simulink model "TSR_Scenario_005_cs"  (Simulink 7.6) (continued)
== system TSR_Scenario_005_cs == (continued)
      mask help:
      | The Actor State contains a vector signal of size (9x1), containing the following signals:
      |
      | -  Position                  (x,y,z)                                     [m]
      | -  Velocity                  (vx,vy,vz)                               [m/s]
      | -  Angles                    (Roll, Pitch, Yaw)                   [rad]
      |
      | The Actor state is defined in the global axis system.
      | The state is superponed on the Initial position defined in the GUI.
      |
      | For more information, please click Help.
      settings: DeleteFcn=PreScanBlockDeleteFcn;
      mask Help: eval('openHelp(222)')
      mask Display: image(imread('State.png'))
  STATE_Toyota_Previa_2 : Subsystem  Tag=State  Priority=0  mask(PreScan Sink Block: State){x0=115.06995391845703, y0=52.373069763183594, z0=0.70999997854232788, Roll0=0, Pitch0=0, Yaw0=0, TimeStep=1/100}
      mask prompts: x0 "Ini x-position VEHICLE wrt GLOBAL axis system [m]"; y0 "Ini y-position VEHICLE wrt GLOBAL axis system [m]"; z0 "Ini z-position VEHICLE wrt GLOBAL axis system [m]"; Roll0 "Ini roll-angle BODY wrt VEHICLE axis system [deg]"; Pitch0 "Ini pitch-angle BODY wrt VEHICLE axis system [deg]"; Yaw0 "Ini yaw-angle VEHICLE wrt GLOBAL axis system [deg]"; TimeStep "Time Step [s]"
      mask help:
      | The Actor State contains a vector signal of size (9x1), containing the following signals:
      |
      | -  Position                  (x,y,z)                                     [m]
      | -  Velocity                  (vx,vy,vz)                               [m/s]
      | -  Angles                    (Roll, Pitch, Yaw)                   [rad]
      |
      | The Actor state is defined in the global axis system.
      | The state is superponed on the Initial position defined in the GUI.
      |
      | For more information, please click Help.
      settings: DeleteFcn=PreScanBlockDeleteFcn;
      mask Help: eval('openHelp(222)')
      mask Display: image(imread('State.png'))
  STATE_Toyota_Previa_3 : Subsystem  Tag=State  Priority=0  mask(PreScan Sink Block: State){x0=94.289756774902344, y0=52.638362884521484, z0=0.70999997854232788, Roll0=0, Pitch0=0, Yaw0=0, TimeStep=1/100}
      mask prompts: x0 "Ini x-position VEHICLE wrt GLOBAL axis system [m]"; y0 "Ini y-position VEHICLE wrt GLOBAL axis system [m]"; z0 "Ini z-position VEHICLE wrt GLOBAL axis system [m]"; Roll0 "Ini roll-angle BODY wrt VEHICLE axis system [deg]"; Pitch0 "Ini pitch-angle BODY wrt VEHICLE axis system [deg]"; Yaw0 "Ini yaw-angle VEHICLE wrt GLOBAL axis system [deg]"; TimeStep "Time Step [s]"
      mask help:
      | The Actor State contains a vector signal of size (9x1), containing the following signals:
      |
      | -  Position                  (x,y,z)                                     [m]
      | -  Velocity                  (vx,vy,vz)                               [m/s]
      | -  Angles                    (Roll, Pitch, Yaw)                   [rad]
      |
      | The Actor state is defined in the global axis system.
      | The state is superponed on the Initial position defined in the GUI.
      |
      | For more information, please click Help.
      settings: DeleteFcn=PreScanBlockDeleteFcn;
      mask Help: eval('openHelp(222)')
      mask Display: image(imread('State.png'))
  STATE_Toyota_Previa_4 : Subsystem  Tag=State  Priority=0  mask(PreScan Sink Block: State){x0=109.62726593017578, y0=90.440689086914063, z0=0.70999997854232788, Roll0=0, Pitch0=0, Yaw0=90, TimeStep=1/100}
      mask prompts: x0 "Ini x-position VEHICLE wrt GLOBAL axis system [m]"; y0 "Ini y-position VEHICLE wrt GLOBAL axis system [m]"; z0 "Ini z-position VEHICLE wrt GLOBAL axis system [m]"; Roll0 "Ini roll-angle BODY wrt VEHICLE axis system [deg]"; Pitch0 "Ini pitch-angle BODY wrt VEHICLE axis system [deg]"; Yaw0 "Ini yaw-angle VEHICLE wrt GLOBAL axis system [deg]"; TimeStep "Time Step [s]"
      mask help:
      | The Actor State contains a vector signal of size (9x1), containing the following signals:
      |
      | -  Position                  (x,y,z)                                     [m]
      | -  Velocity                  (vx,vy,vz)                               [m/s]
      | -  Angles                    (Roll, Pitch, Yaw)                   [rad]
      |
      | The Actor state is defined in the global axis system.
      | The state is superponed on the Initial position defined in the GUI.
      |
      | For more information, please click Help.
      settings: DeleteFcn=PreScanBlockDeleteFcn;
      mask Help: eval('openHelp(222)')
      mask Display: image(imread('State.png'))
  STATE_Toyota_Yaris_1 : Subsystem  Tag=State  Priority=0  mask(PreScan Sink Block: State){x0=144.99999618530273, y0=63.161239624023438, z0=0.57, Roll0=0, Pitch0=0, Yaw0=180, TimeStep=1/100}
      mask prompts: x0 "Ini x-position VEHICLE wrt GLOBAL axis system [m]"; y0 "Ini y-position VEHICLE wrt GLOBAL axis system [m]"; z0 "Ini z-position VEHICLE wrt GLOBAL axis system [m]"; Roll0 "Ini roll-angle BODY wrt VEHICLE axis system [deg]"; Pitch0 "Ini pitch-angle BODY wrt VEHICLE axis system [deg]"; Yaw0 "Ini yaw-angle VEHICLE wrt GLOBAL axis system [deg]"; TimeStep "Time Step [s]"
      mask help:
      | The Actor State contains a vector signal of size (9x1), containing the following signals:
      |
      | -  Position                  (x,y,z)                                     [m]
      | -  Velocity                  (vx,vy,vz)                               [m/s]
      | -  Angles                    (Roll, Pitch, Yaw)                   [rad]
      |
      | The Actor state is defined in the global axis system.
      | The state is superponed on the Initial position defined in the GUI.
      |
      | For more information, please click Help.
      settings: DeleteFcn=PreScanBlockDeleteFcn;
      mask Help: eval('openHelp(222)')
      mask Display: image(imread('State.png'))
  STATE_Toyota_Yaris_2 : Subsystem  Tag=State  Priority=0  mask(PreScan Sink Block: State){x0=318.75335693359375, y0=147.367919921875, z0=0.57, Roll0=0, Pitch0=0, Yaw0=0, TimeStep=1/100}
      mask prompts: x0 "Ini x-position VEHICLE wrt GLOBAL axis system [m]"; y0 "Ini y-position VEHICLE wrt GLOBAL axis system [m]"; z0 "Ini z-position VEHICLE wrt GLOBAL axis system [m]"; Roll0 "Ini roll-angle BODY wrt VEHICLE axis system [deg]"; Pitch0 "Ini pitch-angle BODY wrt VEHICLE axis system [deg]"; Yaw0 "Ini yaw-angle VEHICLE wrt GLOBAL axis system [deg]"; TimeStep "Time Step [s]"
      mask help:
      | The Actor State contains a vector signal of size (9x1), containing the following signals:
      |
      | -  Position                  (x,y,z)                                     [m]
      | -  Velocity                  (vx,vy,vz)                               [m/s]
      | -  Angles                    (Roll, Pitch, Yaw)                   [rad]
      |
      | The Actor state is defined in the global axis system.
      | The state is superponed on the Initial position defined in the GUI.
      |
      | For more information, please click Help.
      settings: DeleteFcn=PreScanBlockDeleteFcn;
      mask Help: eval('openHelp(222)')
      mask Display: image(imread('State.png'))
  SetActuators : Subsystem  Tag=SetActuators  Priority=1  mask(PreScan Simulation Core Block){TimeStep=1/100, NrOfActuators=1661}
      mask prompts: TimeStep "Timestep [s]"; NrOfActuators "Number of actuator values"
      mask help:
      | Please do not remove this block from the PreScan compilation sheet,
      | as it contains essential configuration parameters for a PreScan simulation.
      settings: DeleteFcn=PreScanBlockDeleteFcn;
  SimCoreConfiguration : M-S-Function  Tag=SimCoreConfiguration  Description=128EA0B91E8905FD20CD9FF5500624459A08174AB169DD15EB1AFE5773DF0866  FunctionName=SimCoreBlock  Parameters=ExperimentXML PortsXML SimulationCore HostName CheckStart EnableLog RfR EnableGPUAccel ShowComputationTime  mask(PreScan Simulation Core Configurator){SimulationCore=NUTS, HostName=0, CheckStart=0, prescanversion='7.4.0', EnableLog=off, RfR=0, EnableGPUAccel=off, ShowComputationTime=off}
      mask prompts: SimulationCore "Simulation Core"; HostName "Simulation Host"; CheckStart "Show START Dialog"; prescanversion "PreScan Version"; EnableLog "Create TA Log"; RfR "Record for Replay"; EnableGPUAccel "Enable GPU Acceleration"; ShowComputationTime "Show ComputationTime Scope"
      mask help:
      | Please do not remove this block from the PreScan compilation sheet,
      | as it contains essential configuration parameter for a PreScan simulation.
      mask init:
      | model = bdroot;
      | ExperimentXML = [model(1:end-3) '.pex'];
      | PortsXML = [model '_ports.pcd'];
      settings: DeleteFcn=PreScanBlockDeleteFcn;  ModelCloseFcn=assignin('base',['model_closed_' bdroot],true);
      mask Help: eval('openHelp(812)')
      mask Display: image(imread('prescanlogo.ico'))
  SimCoreInfo : Subsystem  Tag=SimCoreInfo
  Sphere_1 : Subsystem  Tag=WorldObjectSubSystem  InitFcn=UniqueId = '237';
      mask Display: image(imread('Sphere.jpg'));color('white');text(0,0,' ID=68\n')
  Toyota_Previa_1 : Subsystem  Tag=WorldObjectSubSystem  InitFcn=UniqueId = '24';
      mask Display: image(imread('Toyota_Previa.jpg'));color('white');text(0,0,' ID=11\n')
  Toyota_Previa_2 : Subsystem  Tag=WorldObjectSubSystem  InitFcn=UniqueId = '141';
      mask Display: image(imread('Toyota_Previa.jpg'));color('white');text(0,0,' ID=47\n')
  Toyota_Previa_3 : Subsystem  Tag=WorldObjectSubSystem  InitFcn=UniqueId = '142';
      mask Display: image(imread('Toyota_Previa.jpg'));color('white');text(0,0,' ID=48\n')
  Toyota_Previa_4 : Subsystem  Tag=WorldObjectSubSystem  InitFcn=UniqueId = '164';
      mask Display: image(imread('Toyota_Previa.jpg'));color('white');text(0,0,' ID=93\n')
  Toyota_Yaris_1 : Subsystem  Tag=WorldObjectSubSystem  InitFcn=UniqueId = '225';
      mask Display: image(imread('Toyota_Yaris.jpg'));color('white');text(0,0,' ID=32\n')
  Toyota_Yaris_2 : Subsystem  Tag=WorldObjectSubSystem  InitFcn=UniqueId = '610';
      mask Display: image(imread('Toyota_Yaris.jpg'));color('white');text(0,0,' ID=222\n')
  TrafficLightRoadSideNL_1 : Subsystem  Tag=WorldObjectSubSystem  InitFcn=UniqueId = '664';
      mask Display: image(imread('TrafficLightRoadSideNL.jpg'));color('white');text(0,0,' ID=235\n')
  TrafficLightRoadSideNL_2 : Subsystem  Tag=WorldObjectSubSystem  InitFcn=UniqueId = '665';
      mask Display: image(imread('TrafficLightRoadSideNL.jpg'));color('white');text(0,0,' ID=273\n')
  TrafficLightRoadSideNL_3 : Subsystem  Tag=WorldObjectSubSystem  InitFcn=UniqueId = '666';
      mask Display: image(imread('TrafficLightRoadSideNL.jpg'));color('white');text(0,0,' ID=274\n')
  TrafficLightRoadSideNL_4 : Subsystem  Tag=WorldObjectSubSystem  InitFcn=UniqueId = '667';
      mask Display: image(imread('TrafficLightRoadSideNL.jpg'));color('white');text(0,0,' ID=275\n')
  TrafficLightRoadSideNL_5 : Subsystem  Tag=WorldObjectSubSystem  InitFcn=UniqueId = '668';
      mask Display: image(imread('TrafficLightRoadSideNL.jpg'));color('white');text(0,0,' ID=276\n')
  TrafficLightRoadSideNL_6 : Subsystem  Tag=WorldObjectSubSystem  InitFcn=UniqueId = '669';
      mask Display: image(imread('TrafficLightRoadSideNL.jpg'));color('white');text(0,0,' ID=277\n')
  TrafficLightRoadSideNL_7 : Subsystem  Tag=WorldObjectSubSystem  InitFcn=UniqueId = '670';
      mask Display: image(imread('TrafficLightRoadSideNL.jpg'));color('white');text(0,0,' ID=278\n')
  TrafficLightRoadSideNL_8 : Subsystem  Tag=WorldObjectSubSystem  InitFcn=UniqueId = '671';
      mask Display: image(imread('TrafficLightRoadSideNL.jpg'));color('white');text(0,0,' ID=279\n')
  VISUBrakeLightL_Audi_A8_1 : Subsystem  Tag=VisuLights  Priority=0
      settings: DeleteFcn=PreScanBlockDeleteFcn;
      mask Type: PreScan Sink Block: VisuLights
      mask Description:
      | The VisuLights block provides a way to animate the active lights of a vehicle with possibilities to adapt the:
      | - Intensity [-]
      | - Position (x,y,z) [m]
      | - Orientation (Roll, Pitch, Yaw) [deg]
      | - Color (RGB) [-]
      | - TextureResourceID [-]
      | 
      | For more information, please click Help.
      mask Help: eval('openHelp(231)')
      mask Display: image(imread('HeadLight.png'))
  VISUBrakeLightR_Audi_A8_1 : Subsystem  Tag=VisuLights  Priority=0
      settings: DeleteFcn=PreScanBlockDeleteFcn;
      mask Type: PreScan Sink Block: VisuLights
      mask Description:
      | The VisuLights block provides a way to animate the active lights of a vehicle with possibilities to adapt the:
      | - Intensity [-]
      | - Position (x,y,z) [m]
      | - Orientation (Roll, Pitch, Yaw) [deg]
      | - Color (RGB) [-]
      | - TextureResourceID [-]
      | 
      | For more information, please click Help.
      mask Help: eval('openHelp(231)')
      mask Display: image(imread('HeadLight.png'))
  VISUCasingLights_TrafficLightRoadSideNL_1 : Subsystem  Tag=VisuCasingLights  Priority=0
      settings: DeleteFcn=PreScanBlockDeleteFcn;
      mask Type: PreScan Sink Block: VisuLights
      mask Description:
      | The VisuLights block provides a way to animate the indicator and braking lights of a vehicle.
      | 
      | For more information, please click Help.
      mask Help: eval('openHelp(223)')
      mask Display: image(imread('TrafficLightCasing.png'))
  VISUCasingLights_TrafficLightRoadSideNL_2 : Subsystem  Tag=VisuCasingLights  Priority=0
      settings: DeleteFcn=PreScanBlockDeleteFcn;
      mask Type: PreScan Sink Block: VisuLights
      mask Description:
      | The VisuLights block provides a way to animate the indicator and braking lights of a vehicle.
      | 
      | For more information, please click Help.
      mask Help: eval('openHelp(223)')
      mask Display: image(imread('TrafficLightCasing.png'))
  VISUCasingLights_TrafficLightRoadSideNL_3 : Subsystem  Tag=VisuCasingLights  Priority=0
      settings: DeleteFcn=PreScanBlockDeleteFcn;
      mask Type: PreScan Sink Block: VisuLights
      mask Description:
      | The VisuLights block provides a way to animate the indicator and braking lights of a vehicle.
      | 
      | For more information, please click Help.
      mask Help: eval('openHelp(223)')
      mask Display: image(imread('TrafficLightCasing.png'))
  VISUCasingLights_TrafficLightRoadSideNL_4 : Subsystem  Tag=VisuCasingLights  Priority=0
      settings: DeleteFcn=PreScanBlockDeleteFcn;
      mask Type: PreScan Sink Block: VisuLights
      mask Description:
      | The VisuLights block provides a way to animate the indicator and braking lights of a vehicle.
      | 
      | For more information, please click Help.
      mask Help: eval('openHelp(223)')
      mask Display: image(imread('TrafficLightCasing.png'))
  VISUCasingLights_TrafficLightRoadSideNL_5 : Subsystem  Tag=VisuCasingLights  Priority=0
      settings: DeleteFcn=PreScanBlockDeleteFcn;
      mask Type: PreScan Sink Block: VisuLights
      mask Description:
      | The VisuLights block provides a way to animate the indicator and braking lights of a vehicle.
      | 
      | For more information, please click Help.
      mask Help: eval('openHelp(223)')
      mask Display: image(imread('TrafficLightCasing.png'))
  VISUCasingLights_TrafficLightRoadSideNL_6 : Subsystem  Tag=VisuCasingLights  Priority=0
      settings: DeleteFcn=PreScanBlockDeleteFcn;
      mask Type: PreScan Sink Block: VisuLights
      mask Description:
      | The VisuLights block provides a way to animate the indicator and braking lights of a vehicle.
      | 
      | For more information, please click Help.
      mask Help: eval('openHelp(223)')
      mask Display: image(imread('TrafficLightCasing.png'))
  VISUCasingLights_TrafficLightRoadSideNL_7 : Subsystem  Tag=VisuCasingLights  Priority=0
      settings: DeleteFcn=PreScanBlockDeleteFcn;
      mask Type: PreScan Sink Block: VisuLights
      mask Description:
      | The VisuLights block provides a way to animate the indicator and braking lights of a vehicle.
      | 
      | For more information, please click Help.
      mask Help: eval('openHelp(223)')
      mask Display: image(imread('TrafficLightCasing.png'))
  VISUCasingLights_TrafficLightRoadSideNL_8 : Subsystem  Tag=VisuCasingLights  Priority=0
      settings: DeleteFcn=PreScanBlockDeleteFcn;
      mask Type: PreScan Sink Block: VisuLights
      mask Description:
      | The VisuLights block provides a way to animate the indicator and braking lights of a vehicle.
      | 
      | For more information, please click Help.
      mask Help: eval('openHelp(223)')
      mask Display: image(imread('TrafficLightCasing.png'))
  VISUHeadLightLeftHBLB_Audi_A8_1 : Subsystem  Tag=VisuLights  Priority=0
      settings: DeleteFcn=PreScanBlockDeleteFcn;
      mask Type: PreScan Sink Block: VisuLights
      mask Description:
      | The VisuLights block provides a way to animate the active lights of a vehicle with possibilities to adapt the:
      | - Intensity [-]
      | - Position (x,y,z) [m]
      | - Orientation (Roll, Pitch, Yaw) [deg]
      | - Color (RGB) [-]
      | - TextureResourceID [-]
      | 
      | For more information, please click Help.
      mask Help: eval('openHelp(231)')
      mask Display: image(imread('HeadLight.png'))
  VISUHeadLightRightHBLB_Audi_A8_1 : Subsystem  Tag=VisuLights  Priority=0
      settings: DeleteFcn=PreScanBlockDeleteFcn;
      mask Type: PreScan Sink Block: VisuLights
      mask Description:
      | The VisuLights block provides a way to animate the active lights of a vehicle with possibilities to adapt the:
      | - Intensity [-]
      | - Position (x,y,z) [m]
      | - Orientation (Roll, Pitch, Yaw) [deg]
      | - Color (RGB) [-]
      | - TextureResourceID [-]
      | 
      | For more information, please click Help.
      mask Help: eval('openHelp(231)')
      mask Display: image(imread('HeadLight.png'))
  VISULightPostLight_Lightpost_1 : Subsystem  Tag=VisuLights  Priority=0
      settings: DeleteFcn=PreScanBlockDeleteFcn;
      mask Type: PreScan Sink Block: VisuLights
      mask Description:
      | The VisuLights block provides a way to animate the active lights of a vehicle with possibilities to adapt the:
      | - Intensity [-]
      | - Position (x,y,z) [m]
      | - Orientation (Roll, Pitch, Yaw) [deg]
      | - Color (RGB) [-]
      | - TextureResourceID [-]
      | 
      | For more information, please click Help.
      mask Help: eval('openHelp(231)')
      mask Display: image(imread('HeadLight.png'))
  VISULightPostLight_Lightpost_11 : Subsystem  Tag=VisuLights  Priority=0
      settings: DeleteFcn=PreScanBlockDeleteFcn;
      mask Type: PreScan Sink Block: VisuLights
      mask Description:
      | The VisuLights block provides a way to animate the active lights of a vehicle with possibilities to adapt the:
      | - Intensity [-]
      | - Position (x,y,z) [m]
      | - Orientation (Roll, Pitch, Yaw) [deg]
      | - Color (RGB) [-]
      | - TextureResourceID [-]
      | 
      | For more information, please click Help.
      mask Help: eval('openHelp(231)')
      mask Display: image(imread('HeadLight.png'))
  VISULightPostLight_Lightpost_12 : Subsystem  Tag=VisuLights  Priority=0
      settings: DeleteFcn=PreScanBlockDeleteFcn;
      mask Type: PreScan Sink Block: VisuLights
      mask Description:
      | The VisuLights block provides a way to animate the active lights of a vehicle with possibilities to adapt the:
      | - Intensity [-]
      | - Position (x,y,z) [m]
      | - Orientation (Roll, Pitch, Yaw) [deg]
      | - Color (RGB) [-]
      | - TextureResourceID [-]
      | 
      | For more information, please click Help.
      mask Help: eval('openHelp(231)')
      mask Display: image(imread('HeadLight.png'))
  VISULightPostLight_Lightpost_2 : Subsystem  Tag=VisuLights  Priority=0
      settings: DeleteFcn=PreScanBlockDeleteFcn;
      mask Type: PreScan Sink Block: VisuLights
      mask Description:
      | The VisuLights block provides a way to animate the active lights of a vehicle with possibilities to adapt the:
      | - Intensity [-]
      | - Position (x,y,z) [m]
      | - Orientation (Roll, Pitch, Yaw) [deg]
      | - Color (RGB) [-]
      | - TextureResourceID [-]
      | 
      | For more information, please click Help.
      mask Help: eval('openHelp(231)')
      mask Display: image(imread('HeadLight.png'))
  VISULightPostLight_Lightpost_25 : Subsystem  Tag=VisuLights  Priority=0
      settings: DeleteFcn=PreScanBlockDeleteFcn;
      mask Type: PreScan Sink Block: VisuLights
      mask Description:
      | The VisuLights block provides a way to animate the active lights of a vehicle with possibilities to adapt the:
      | - Intensity [-]
      | - Position (x,y,z) [m]
      | - Orientation (Roll, Pitch, Yaw) [deg]
      | - Color (RGB) [-]
      | - TextureResourceID [-]
      | 
      | For more information, please click Help.
      mask Help: eval('openHelp(231)')
      mask Display: image(imread('HeadLight.png'))
  VISULightPostLight_Lightpost_27 : Subsystem  Tag=VisuLights  Priority=0
      settings: DeleteFcn=PreScanBlockDeleteFcn;
      mask Type: PreScan Sink Block: VisuLights
      mask Description:
      | The VisuLights block provides a way to animate the active lights of a vehicle with possibilities to adapt the:
      | - Intensity [-]
      | - Position (x,y,z) [m]
      | - Orientation (Roll, Pitch, Yaw) [deg]
      | - Color (RGB) [-]
      | - TextureResourceID [-]
      | 
      | For more information, please click Help.
      mask Help: eval('openHelp(231)')
      mask Display: image(imread('HeadLight.png'))
  VISULightPostLight_Lightpost_3 : Subsystem  Tag=VisuLights  Priority=0
      settings: DeleteFcn=PreScanBlockDeleteFcn;
      mask Type: PreScan Sink Block: VisuLights
      mask Description:
      | The VisuLights block provides a way to animate the active lights of a vehicle with possibilities to adapt the:
      | - Intensity [-]
      | - Position (x,y,z) [m]
      | - Orientation (Roll, Pitch, Yaw) [deg]
      | - Color (RGB) [-]
      | - TextureResourceID [-]
      | 
      | For more information, please click Help.
      mask Help: eval('openHelp(231)')
      mask Display: image(imread('HeadLight.png'))
  VISULightPostLight_Lightpost_34 : Subsystem  Tag=VisuLights  Priority=0
      settings: DeleteFcn=PreScanBlockDeleteFcn;
      mask Type: PreScan Sink Block: VisuLights
      mask Description:
      | The VisuLights block provides a way to animate the active lights of a vehicle with possibilities to adapt the:
      | - Intensity [-]
      | - Position (x,y,z) [m]
      | - Orientation (Roll, Pitch, Yaw) [deg]
      | - Color (RGB) [-]
      | - TextureResourceID [-]
      | 
      | For more information, please click Help.
      mask Help: eval('openHelp(231)')
      mask Display: image(imread('HeadLight.png'))
  VISULightPostLight_Lightpost_36 : Subsystem  Tag=VisuLights  Priority=0
      settings: DeleteFcn=PreScanBlockDeleteFcn;
      mask Type: PreScan Sink Block: VisuLights
      mask Description:
      | The VisuLights block provides a way to animate the active lights of a vehicle with possibilities to adapt the:
      | - Intensity [-]
      | - Position (x,y,z) [m]
      | - Orientation (Roll, Pitch, Yaw) [deg]
      | - Color (RGB) [-]
      | - TextureResourceID [-]
      | 
      | For more information, please click Help.
      mask Help: eval('openHelp(231)')
      mask Display: image(imread('HeadLight.png'))
  VISULightPostLight_Lightpost_38 : Subsystem  Tag=VisuLights  Priority=0
      settings: DeleteFcn=PreScanBlockDeleteFcn;
      mask Type: PreScan Sink Block: VisuLights
      mask Description:
      | The VisuLights block provides a way to animate the active lights of a vehicle with possibilities to adapt the:
      | - Intensity [-]
      | - Position (x,y,z) [m]
      | - Orientation (Roll, Pitch, Yaw) [deg]
      | - Color (RGB) [-]
      | - TextureResourceID [-]
      | 
      | For more information, please click Help.
      mask Help: eval('openHelp(231)')
      mask Display: image(imread('HeadLight.png'))
  VISULightPostLight_Lightpost_4 : Subsystem  Tag=VisuLights  Priority=0
      settings: DeleteFcn=PreScanBlockDeleteFcn;
      mask Type: PreScan Sink Block: VisuLights
      mask Description:
      | The VisuLights block provides a way to animate the active lights of a vehicle with possibilities to adapt the:
      | - Intensity [-]
      | - Position (x,y,z) [m]
      | - Orientation (Roll, Pitch, Yaw) [deg]
      | - Color (RGB) [-]
      | - TextureResourceID [-]
      | 
      | For more information, please click Help.
      mask Help: eval('openHelp(231)')
      mask Display: image(imread('HeadLight.png'))
  VISULightPostLight_Lightpost_40 : Subsystem  Tag=VisuLights  Priority=0
      settings: DeleteFcn=PreScanBlockDeleteFcn;
      mask Type: PreScan Sink Block: VisuLights
      mask Description:
      | The VisuLights block provides a way to animate the active lights of a vehicle with possibilities to adapt the:
      | - Intensity [-]
      | - Position (x,y,z) [m]
      | - Orientation (Roll, Pitch, Yaw) [deg]
      | - Color (RGB) [-]
      | - TextureResourceID [-]
      | 
      | For more information, please click Help.
      mask Help: eval('openHelp(231)')
      mask Display: image(imread('HeadLight.png'))
  VISULightPostLight_Lightpost_42 : Subsystem  Tag=VisuLights  Priority=0
      settings: DeleteFcn=PreScanBlockDeleteFcn;
      mask Type: PreScan Sink Block: VisuLights
      mask Description:
      | The VisuLights block provides a way to animate the active lights of a vehicle with possibilities to adapt the:
      | - Intensity [-]
      | - Position (x,y,z) [m]
      | - Orientation (Roll, Pitch, Yaw) [deg]
      | - Color (RGB) [-]
      | - TextureResourceID [-]
      | 
      | For more information, please click Help.
      mask Help: eval('openHelp(231)')
      mask Display: image(imread('HeadLight.png'))
  VISULightPostLight_Lightpost_44 : Subsystem  Tag=VisuLights  Priority=0
      settings: DeleteFcn=PreScanBlockDeleteFcn;
      mask Type: PreScan Sink Block: VisuLights
      mask Description:
      | The VisuLights block provides a way to animate the active lights of a vehicle with possibilities to adapt the:
      | - Intensity [-]
      | - Position (x,y,z) [m]
      | - Orientation (Roll, Pitch, Yaw) [deg]
      | - Color (RGB) [-]
      | - TextureResourceID [-]
      | 
      | For more information, please click Help.
      mask Help: eval('openHelp(231)')
      mask Display: image(imread('HeadLight.png'))
  VISULightPostLight_Lightpost_5 : Subsystem  Tag=VisuLights  Priority=0
      settings: DeleteFcn=PreScanBlockDeleteFcn;
      mask Type: PreScan Sink Block: VisuLights
      mask Description:
      | The VisuLights block provides a way to animate the active lights of a vehicle with possibilities to adapt the:
      | - Intensity [-]
      | - Position (x,y,z) [m]
      | - Orientation (Roll, Pitch, Yaw) [deg]
      | - Color (RGB) [-]
      | - TextureResourceID [-]
      | 
      | For more information, please click Help.
      mask Help: eval('openHelp(231)')
      mask Display: image(imread('HeadLight.png'))
  VISULightPostLight_Lightpost_54 : Subsystem  Tag=VisuLights  Priority=0
      settings: DeleteFcn=PreScanBlockDeleteFcn;
      mask Type: PreScan Sink Block: VisuLights
      mask Description:
      | The VisuLights block provides a way to animate the active lights of a vehicle with possibilities to adapt the:
      | - Intensity [-]
      | - Position (x,y,z) [m]
      | - Orientation (Roll, Pitch, Yaw) [deg]
      | - Color (RGB) [-]
      | - TextureResourceID [-]
      | 
      | For more information, please click Help.
      mask Help: eval('openHelp(231)')
      mask Display: image(imread('HeadLight.png'))
  VISULightSource_Audi_A8_1 : Subsystem  Tag=VisuLightSource  Priority=0
      settings: DeleteFcn=PreScanBlockDeleteFcn;
      mask Type: PreScan Sink Block: VisuLightSource
      mask Description:
      | The VisuLightSource block provides a way to animate Light Sources of the indicator, braking, fog and main lights of a vehicle.
      | 
      | For more information, please click Help.
      mask Help: eval('openHelp(223)')
      mask Display: image(imread('RearLight.png'))
  VISUSteer_Audi_A8_1 : Subsystem  Tag=VisuSteer  Priority=0
      settings: DeleteFcn=PreScanBlockDeleteFcn;
      mask Type: PreScan Sink Block: VisuSteer
      mask Description:
      | The VisuSteer block provides a way to adapt the orientation of the steering column and steering wheel of a vehicle.
      | 
      | For more information, please click Help.
      mask Help: eval('openHelp(223)')
      mask Display: image(imread('steeringwheel.png'))
  VISUTextureColor_Fiat_Bravo_1 : Subsystem  Tag=VisuTextureColor  Priority=0
      settings: DeleteFcn=PreScanBlockDeleteFcn;
      mask Type: PreScan Sink Block: VisuTextureColor
      mask Description:
      | The Texture Color block provides a way to adapt the color of a vehicle.
      | 
      | For more information, please click Help.
      mask Help: eval('openHelp(230)')
      mask Display: image(imread('TextureColor.png'))
  VISUTextureColor_Toyota_Previa_1 : Subsystem  Tag=VisuTextureColor  Priority=0
      settings: DeleteFcn=PreScanBlockDeleteFcn;
      mask Type: PreScan Sink Block: VisuTextureColor
      mask Description:
      | The Texture Color block provides a way to adapt the color of a vehicle.
      | 
      | For more information, please click Help.
      mask Help: eval('openHelp(230)')
      mask Display: image(imread('TextureColor.png'))
  VISUWheels_Audi_A8_1 : Subsystem  Tag=VisuWheels  Priority=0
      settings: DeleteFcn=PreScanBlockDeleteFcn;
      mask Type: PreScan Sink Block: VisuWheels
      mask Description:
      | The VisuWheels block provides a way to animate the wheels of a vehicle, with steering, rotation (because of velocity) and z-displacement.
      | 
      | For more information, please click Help.
      mask Help: eval('openHelp(223)')
      mask Display: image(imread('wheel.png'))
  VISUWheels_Ford_Fiesta_1 : Subsystem  Tag=VisuWheels  Priority=0
      settings: DeleteFcn=PreScanBlockDeleteFcn;
      mask Type: PreScan Sink Block: VisuWheels
      mask Description:
      | The VisuWheels block provides a way to animate the wheels of a vehicle, with steering, rotation (because of velocity) and z-displacement.
      | 
      | For more information, please click Help.
      mask Help: eval('openHelp(223)')
      mask Display: image(imread('wheel.png'))
  VISUWheels_Toyota_Yaris_1 : Subsystem  Tag=VisuWheels  Priority=0
      settings: DeleteFcn=PreScanBlockDeleteFcn;
      mask Type: PreScan Sink Block: VisuWheels
      mask Description:
      | The VisuWheels block provides a way to animate the wheels of a vehicle, with steering, rotation (because of velocity) and z-displacement.
      | 
      | For more information, please click Help.
      mask Help: eval('openHelp(223)')
      mask Display: image(imread('wheel.png'))
  wires:
  BMW_X5_1/1(STATE_BMW_X5_1) -> STATE_BMW_X5_1/1(State)
  SELF_BMW_X5_1/1(U) -> BMW_X5_1/1(SELF_BMW_X5_1)
  BMW_Z3_1/1(STATE_BMW_Z3_1) -> STATE_BMW_Z3_1/1(State)
  SELF_BMW_Z3_1/1(U) -> BMW_Z3_1/1(SELF_BMW_Z3_1)
  Toyota_Previa_1/1(STATE_Toyota_Previa_1) -> STATE_Toyota_Previa_1/1(State)
  SELF_Toyota_Previa_1/1(U) -> Toyota_Previa_1/1(SELF_Toyota_Previa_1)
  Toyota_Previa_1/2(VISUTextureColor_Toyota_Previa_1) -> VISUTextureColor_Toyota_Previa_1/1(TextureColor)
  Nissan_Cabstar_1/1(STATE_Nissan_Cabstar_1) -> STATE_Nissan_Cabstar_1/1(State)
  SELF_Nissan_Cabstar_1/1(U) -> Nissan_Cabstar_1/1(SELF_Nissan_Cabstar_1)
  Mazda_RX8_2/1(STATE_Mazda_RX8_2) -> STATE_Mazda_RX8_2/1(State)
  SELF_Mazda_RX8_2/1(U) -> Mazda_RX8_2/1(SELF_Mazda_RX8_2)
  Fiat_Bravo_2/1(STATE_Fiat_Bravo_2) -> STATE_Fiat_Bravo_2/1(State)
  SELF_Fiat_Bravo_2/1(U) -> Fiat_Bravo_2/1(SELF_Fiat_Bravo_2)
  Toyota_Previa_2/1(STATE_Toyota_Previa_2) -> STATE_Toyota_Previa_2/1(State)
  SELF_Toyota_Previa_2/1(U) -> Toyota_Previa_2/1(SELF_Toyota_Previa_2)
  Toyota_Previa_3/1(STATE_Toyota_Previa_3) -> STATE_Toyota_Previa_3/1(State)
  SELF_Toyota_Previa_3/1(U) -> Toyota_Previa_3/1(SELF_Toyota_Previa_3)
  Mazda_RX8_3/1(STATE_Mazda_RX8_3) -> STATE_Mazda_RX8_3/1(State)
  SELF_Mazda_RX8_3/1(U) -> Mazda_RX8_3/1(SELF_Mazda_RX8_3)
  Nissan_Cabstar_2/1(STATE_Nissan_Cabstar_2) -> STATE_Nissan_Cabstar_2/1(State)
  SELF_Nissan_Cabstar_2/1(U) -> Nissan_Cabstar_2/1(SELF_Nissan_Cabstar_2)
  Toyota_Previa_4/1(STATE_Toyota_Previa_4) -> STATE_Toyota_Previa_4/1(State)
  SELF_Toyota_Previa_4/1(U) -> Toyota_Previa_4/1(SELF_Toyota_Previa_4)
  BMW_X5_2/1(STATE_BMW_X5_2) -> STATE_BMW_X5_2/1(State)
  SELF_BMW_X5_2/1(U) -> BMW_X5_2/1(SELF_BMW_X5_2)
  Audi_A8_1/1(STATE_Audi_A8_1) -> STATE_Audi_A8_1/1(State)
  SELF_Audi_A8_1/1(U) -> Audi_A8_1/1(SELF_Audi_A8_1)
  CameraSensor_1/1(U1) -> Audi_A8_1/2(CameraSensor_1)
  Audi_A8_1/2(VISULightSource_Audi_A8_1) -> VISULightSource_Audi_A8_1/1(Lights)
  Audi_A8_1/3(VISUWheels_Audi_A8_1) -> VISUWheels_Audi_A8_1/1(Wheels)
  Audi_A8_1/4(VISUSteer_Audi_A8_1) -> VISUSteer_Audi_A8_1/1(Steer)
  Audi_A8_1/5(VISUHeadLightRightHBLB_Audi_A8_1) -> VISUHeadLightRightHBLB_Audi_A8_1/1(Lights)
  Audi_A8_1/6(VISUHeadLightLeftHBLB_Audi_A8_1) -> VISUHeadLightLeftHBLB_Audi_A8_1/1(Lights)
  Audi_A8_1/7(VISUBrakeLightL_Audi_A8_1) -> VISUBrakeLightL_Audi_A8_1/1(Lights)
  Audi_A8_1/8(VISUBrakeLightR_Audi_A8_1) -> VISUBrakeLightR_Audi_A8_1/1(Lights)
  Toyota_Yaris_1/1(STATE_Toyota_Yaris_1) -> STATE_Toyota_Yaris_1/1(State)
  SELF_Toyota_Yaris_1/1(U) -> Toyota_Yaris_1/1(SELF_Toyota_Yaris_1)
  Toyota_Yaris_1/2(VISUWheels_Toyota_Yaris_1) -> VISUWheels_Toyota_Yaris_1/1(Wheels)
  Ford_Fiesta_1/1(STATE_Ford_Fiesta_1) -> STATE_Ford_Fiesta_1/1(State)
  SELF_Ford_Fiesta_1/1(U) -> Ford_Fiesta_1/1(SELF_Ford_Fiesta_1)
  Ford_Fiesta_1/2(VISUWheels_Ford_Fiesta_1) -> VISUWheels_Ford_Fiesta_1/1(Wheels)
  CarTrailer_SingleAxle_1/1(STATE_CarTrailer_SingleAxle_1) -> STATE_CarTrailer_SingleAxle_1/1(State)
  SELF_CarTrailer_SingleAxle_1/1(U) -> CarTrailer_SingleAxle_1/1(SELF_CarTrailer_SingleAxle_1)
  Sphere_1/1(STATE_Sphere_1) -> STATE_Sphere_1/1(State)
  SELF_Sphere_1/1(U) -> Sphere_1/1(SELF_Sphere_1)
  Fiat_Bravo_1/1(STATE_Fiat_Bravo_1) -> STATE_Fiat_Bravo_1/1(State)
  SELF_Fiat_Bravo_1/1(U) -> Fiat_Bravo_1/1(SELF_Fiat_Bravo_1)
  Fiat_Bravo_1/2(VISUTextureColor_Fiat_Bravo_1) -> VISUTextureColor_Fiat_Bravo_1/1(TextureColor)
  Male_RegularAni_1/1(STATE_Male_RegularAni_1) -> STATE_Male_RegularAni_1/1(State)
  SELF_Male_RegularAni_1/1(U) -> Male_RegularAni_1/1(SELF_Male_RegularAni_1)
  Female_Regular_wBuggy_1/1(STATE_Female_Regular_wBuggy_1) -> STATE_Female_Regular_wBuggy_1/1(State)
  SELF_Female_Regular_wBuggy_1/1(U) -> Female_Regular_wBuggy_1/1(SELF_Female_Regular_wBuggy_1)
  Male_Regular_wBicycle_1/1(STATE_Male_Regular_wBicycle_1) -> STATE_Male_Regular_wBicycle_1/1(State)
  SELF_Male_Regular_wBicycle_1/1(U) -> Male_Regular_wBicycle_1/1(SELF_Male_Regular_wBicycle_1)
  Citroen_C3_1/1(STATE_Citroen_C3_1) -> STATE_Citroen_C3_1/1(State)
  SELF_Citroen_C3_1/1(U) -> Citroen_C3_1/1(SELF_Citroen_C3_1)
  BMW_X5_3/1(STATE_BMW_X5_3) -> STATE_BMW_X5_3/1(State)
  SELF_BMW_X5_3/1(U) -> BMW_X5_3/1(SELF_BMW_X5_3)
  Male_Regular_wSuitcase_1/1(STATE_Male_Regular_wSuitcase_1) -> STATE_Male_Regular_wSuitcase_1/1(State)
  SELF_Male_Regular_wSuitcase_1/1(U) -> Male_Regular_wSuitcase_1/1(SELF_Male_Regular_wSuitcase_1)
  Actros_2541_1/1(STATE_Actros_2541_1) -> STATE_Actros_2541_1/1(State)
  SELF_Actros_2541_1/1(U) -> Actros_2541_1/1(SELF_Actros_2541_1)
  FullCurtainSidedTrailer_1/1(STATE_FullCurtainSidedTrailer_1) -> STATE_FullCurtainSidedTrailer_1/1(State)
  SELF_FullCurtainSidedTrailer_1/1(U) -> FullCurtainSidedTrailer_1/1(SELF_FullCurtainSidedTrailer_1)
  Daf_95_1/1(STATE_Daf_95_1) -> STATE_Daf_95_1/1(State)
  SELF_Daf_95_1/1(U) -> Daf_95_1/1(SELF_Daf_95_1)
  Female_RegularAni_1/1(STATE_Female_RegularAni_1) -> STATE_Female_RegularAni_1/1(State)
  SELF_Female_RegularAni_1/1(U) -> Female_RegularAni_1/1(SELF_Female_RegularAni_1)
  Male_Regular_wBackpack_1/1(STATE_Male_Regular_wBackpack_1) -> STATE_Male_Regular_wBackpack_1/1(State)
  SELF_Male_Regular_wBackpack_1/1(U) -> Male_Regular_wBackpack_1/1(SELF_Male_Regular_wBackpack_1)
  Honda_Pan_European_1/1(STATE_Honda_Pan_European_1) -> STATE_Honda_Pan_European_1/1(State)
  SELF_Honda_Pan_European_1/1(U) -> Honda_Pan_European_1/1(SELF_Honda_Pan_European_1)
  Toyota_Yaris_2/1(STATE_Toyota_Yaris_2) -> STATE_Toyota_Yaris_2/1(State)
  SELF_Toyota_Yaris_2/1(U) -> Toyota_Yaris_2/1(SELF_Toyota_Yaris_2)
  Mazda_RX8_1/1(STATE_Mazda_RX8_1) -> STATE_Mazda_RX8_1/1(State)
  SELF_Mazda_RX8_1/1(U) -> Mazda_RX8_1/1(SELF_Mazda_RX8_1)
  Actros_1860_1/1(STATE_Actros_1860_1) -> STATE_Actros_1860_1/1(State)
  SELF_Actros_1860_1/1(U) -> Actros_1860_1/1(SELF_Actros_1860_1)
  Lightpost_2/1(VISULightPostLight_Lightpost_2) -> VISULightPostLight_Lightpost_2/1(Lights)
  Lightpost_1/1(VISULightPostLight_Lightpost_1) -> VISULightPostLight_Lightpost_1/1(Lights)
  Lightpost_3/1(VISULightPostLight_Lightpost_3) -> VISULightPostLight_Lightpost_3/1(Lights)
  Lightpost_4/1(VISULightPostLight_Lightpost_4) -> VISULightPostLight_Lightpost_4/1(Lights)
  Lightpost_5/1(VISULightPostLight_Lightpost_5) -> VISULightPostLight_Lightpost_5/1(Lights)
  Lightpost_11/1(VISULightPostLight_Lightpost_11) -> VISULightPostLight_Lightpost_11/1(Lights)
  Lightpost_12/1(VISULightPostLight_Lightpost_12) -> VISULightPostLight_Lightpost_12/1(Lights)
  Lightpost_25/1(VISULightPostLight_Lightpost_25) -> VISULightPostLight_Lightpost_25/1(Lights)
  Lightpost_27/1(VISULightPostLight_Lightpost_27) -> VISULightPostLight_Lightpost_27/1(Lights)
  Lightpost_34/1(VISULightPostLight_Lightpost_34) -> VISULightPostLight_Lightpost_34/1(Lights)
  Lightpost_36/1(VISULightPostLight_Lightpost_36) -> VISULightPostLight_Lightpost_36/1(Lights)
  Lightpost_38/1(VISULightPostLight_Lightpost_38) -> VISULightPostLight_Lightpost_38/1(Lights)
  Lightpost_40/1(VISULightPostLight_Lightpost_40) -> VISULightPostLight_Lightpost_40/1(Lights)
  Lightpost_42/1(VISULightPostLight_Lightpost_42) -> VISULightPostLight_Lightpost_42/1(Lights)
  Lightpost_44/1(VISULightPostLight_Lightpost_44) -> VISULightPostLight_Lightpost_44/1(Lights)
  TrafficLightRoadSideNL_1/1(VISUCasingLights_TrafficLightRoadSideNL_1) -> VISUCasingLights_TrafficLightRoadSideNL_1/1(CasingLights)
  TrafficLightRoadSideNL_2/1(VISUCasingLights_TrafficLightRoadSideNL_2) -> VISUCasingLights_TrafficLightRoadSideNL_2/1(CasingLights)
  TrafficLightRoadSideNL_3/1(VISUCasingLights_TrafficLightRoadSideNL_3) -> VISUCasingLights_TrafficLightRoadSideNL_3/1(CasingLights)
  TrafficLightRoadSideNL_4/1(VISUCasingLights_TrafficLightRoadSideNL_4) -> VISUCasingLights_TrafficLightRoadSideNL_4/1(CasingLights)
  TrafficLightRoadSideNL_5/1(VISUCasingLights_TrafficLightRoadSideNL_5) -> VISUCasingLights_TrafficLightRoadSideNL_5/1(CasingLights)
  TrafficLightRoadSideNL_6/1(VISUCasingLights_TrafficLightRoadSideNL_6) -> VISUCasingLights_TrafficLightRoadSideNL_6/1(CasingLights)
  TrafficLightRoadSideNL_7/1(VISUCasingLights_TrafficLightRoadSideNL_7) -> VISUCasingLights_TrafficLightRoadSideNL_7/1(CasingLights)
  TrafficLightRoadSideNL_8/1(VISUCasingLights_TrafficLightRoadSideNL_8) -> VISUCasingLights_TrafficLightRoadSideNL_8/1(CasingLights)
  Lightpost_54/1(VISULightPostLight_Lightpost_54) -> VISULightPostLight_Lightpost_54/1(Lights)
  SetActuators/1(dummy) -> GetSensors/1(dummy)
